simulink model "demo_fengine"  (Simulink 7.4)
settings: Solver=ode45  StartTime=0.0  StopTime=10.0  RelTol=1e-3
undefined symbols (not defined in the file): pfb_coeff_gen_calc
libraries: xbsIndex_r4 x5167, casper_library x437, isi_correlator_lib x15, xps_library x7
(part 1 of 50)
settings Model: Creator=wjm  LastModifiedBy=wjm

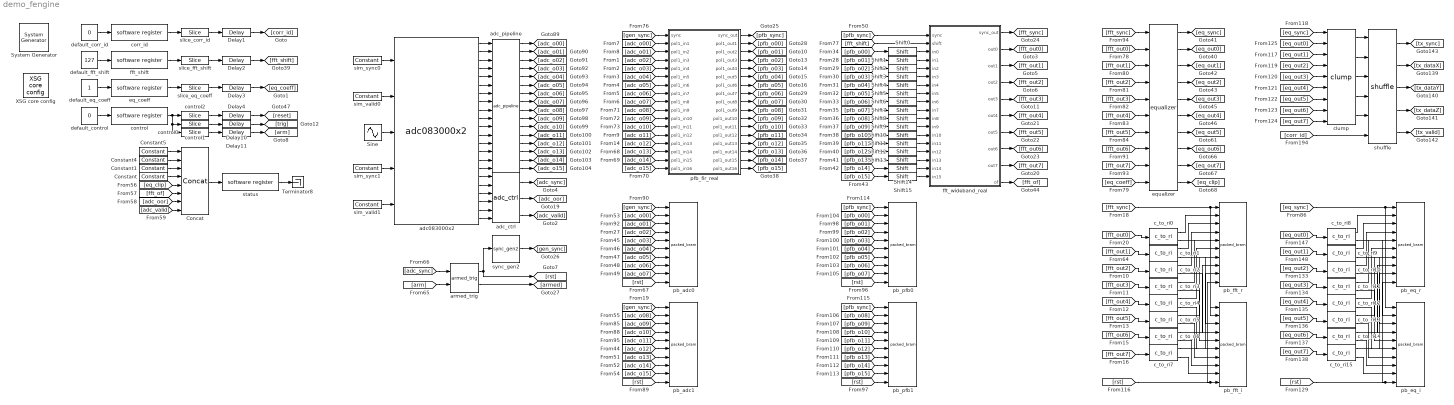
== system demo_fengine ==
  " System Generator" : xbsIndex_r4/ System Generator  Tag=genX  SystemSampleTime=-1  xilinxfamily=virtex5  part=xc5vsx95t  speed=-1  package=ff1136  synthesis_tool=XST  clock_wrapper=Clock Enables  directory=./demo_fengine/sysgen  testbench=off  simulink_period=1  sysclk_period=5  dcm_input_clock_period=10  incr_netlist=off  trim_vbits=Everywhere in SubSystem  dbl_ovrd=According to Block Masks  core_generation=According to Block Masks  run_coregen=off  deprecated_control=off  eval_field=0  block_type=sysgen
      settings: block_version=11.3
  Concat : xbsIndex_r4/Concat  num_inputs=8  dbl_ovrd=off  block_type=concat
      settings: block_version=10.1.3
  Constant{,1,4..5} (4 alike) : xbsIndex_r4/Constant  arith_type=Boolean  const=0  n_bits=1  bin_pt=0  explicit_period=on  period=1  equ=P=C  opselect=C  inp2=PCIN>>17  opr=+  inp1=P  carry=CIN  dbl_ovrd=off  block_type=constant
      settings: block_version=10.1.1
  Delay{1..4,10..11} (6 alike) : xbsIndex_r4/Delay  en=off  latency=1  dbl_ovrd=off  reg_retiming=on  block_type=delay
  Shift{0..15} (16 alike) : xbsIndex_r4/Shift  shift_dir=Right  shift_bits=1  en=off  latency=0  precision=Full  arith_type="Signed  (2's comp)"  n_bits=16  bin_pt=14  quantization=Truncate  overflow=Wrap  dbl_ovrd=off  block_type=shift
  Sine : Sin  Amplitude=.9  Bias=0  Frequency=37.5*(2*pi/200)  Phase=0  Samples=10  SampleTime=1/16  Offset=0
  Terminator8 : Terminator
  XSG core config : xps_library/XSG core config  Tag=xps:xsg  SystemSampleTime=-1  hw_sys=ROACH:sx95t  ibob_linux=off  clk_src=adc0_clk  iBOB_clk_src=sys_clk  BEE2_ctrl_clk_src=sys_clk  BEE2_usr_clk_src=sys_clk  ROACH_clk_src=adc0_clk  CORR_clk_src=sys_clk  gpio_clk_io_group=iBOB:sma  gpio_clk_bit_index=0  clk_rate=200  sample_period=1  synthesis_tool=XST  mpc_type=powerpc440_ext
  adc083000x2 : xps_library/adc083000x2  Tag=xps:adc083000x2  SystemSampleTime=-1  use_adc0=on  use_adc1=on  demux_adc=off  clock_sync=on  verbose=off  using_ctrl=off  adc_clk_rate=800  sample_period=1  bit_width=8
  adc_ctrl : isi_correlator_lib/adc_ctrl  SystemSampleTime=-1
  adc_pipeline : isi_correlator_lib/adc_pipeline  SystemSampleTime=-1  latency=1
  armed_trig : isi_correlator_lib/armed_trig  SystemSampleTime=-1
  c_to_ri{0..7} (8 alike) : casper_library/Misc/c_to_ri  SystemSampleTime=-1  n_bits=18  bin_pt=17
      settings: AttributesFormatString=18_17 r/i
  c_to_ri{8..15} (8 alike) : casper_library/Misc/c_to_ri  SystemSampleTime=-1  n_bits=4  bin_pt=3
      settings: AttributesFormatString=4_3 r/i
  clump : isi_correlator_lib/clump  SystemSampleTime=-1  latency=1
  control : xps_library/software register  Tag=xps:sw_reg  SystemSampleTime=-1  io_dir=From Processor  arith_type=Unsigned  bitwidth=32  bin_pt=0  sample_period=1  gw_name=demo_fengine_control_user_data_out
  control0 : xbsIndex_r4/Slice  nbits=1  boolean_output=on  mode=Lower Bit Location + Width  bit1=0  base1=MSB of Input  bit0=0  base0=LSB of Input  dbl_ovrd=off  block_type=slice
      settings: infoedit="Extracts a given range of bits from each input sample and presents it at the output.  The output type is ordinarily unsigned with binary point at zero, but can be Boolean when the slice is one bit wide.<P><P>Hardware notes: In hardware this block costs nothing."  block_version=10.1.3
  control1 : xbsIndex_r4/Slice  nbits=1  boolean_output=on  mode=Lower Bit Location + Width  bit1=0  base1=MSB of Input  bit0=1  base0=LSB of Input  dbl_ovrd=off  block_type=slice
      settings: infoedit="Extracts a given range of bits from each input sample and presents it at the output.  The output type is ordinarily unsigned with binary point at zero, but can be Boolean when the slice is one bit wide.<P><P>Hardware notes: In hardware this block costs nothing."  block_version=10.1.3
  control2 : xbsIndex_r4/Slice  nbits=1  boolean_output=on  mode=Lower Bit Location + Width  bit1=0  base1=MSB of Input  bit0=2  base0=LSB of Input  dbl_ovrd=off  block_type=slice
      settings: infoedit="Extracts a given range of bits from each input sample and presents it at the output.  The output type is ordinarily unsigned with binary point at zero, but can be Boolean when the slice is one bit wide.<P><P>Hardware notes: In hardware this block costs nothing."  block_version=10.1.3
  corr_id : xps_library/software register  Tag=xps:sw_reg  SystemSampleTime=-1  io_dir=From Processor  arith_type=Unsigned  bitwidth=32  bin_pt=0  sample_period=1  gw_name=demo_fengine_corr_id_user_data_out
  default_control : Constant  Value=0  OutDataType=sfix(16)  OutScaling=2^0  SampleTime=1
  default_corr_id : Constant  Value=0  OutDataType=sfix(16)  OutScaling=2^0  SampleTime=1
  default_eq_coeff : Constant  OutDataType=sfix(16)  OutScaling=2^0  SampleTime=1
  default_fft_shift : Constant  Value=127  OutDataType=sfix(16)  OutScaling=2^0  SampleTime=1
  eq_coeff : xps_library/software register  Tag=xps:sw_reg  SystemSampleTime=-1  io_dir=From Processor  arith_type=Unsigned  bitwidth=32  bin_pt=0  sample_period=1  gw_name=demo_fengine_eq_coeff_user_data_out
  equalizer : isi_correlator_lib/equalizer  SystemSampleTime=-1  in_bit_width=18  out_bit_width=4
  fft_shift : xps_library/software register  Tag=xps:sw_reg  SystemSampleTime=-1  io_dir=From Processor  arith_type=Unsigned  bitwidth=32  bin_pt=0  sample_period=1  gw_name=demo_fengine_fft_shift_user_data_out
  fft_wideband_real : Subsystem  AncestorBlock=casper_library/FFTs/fft_wideband_real  mask(fft_wideband_real){FFTSize=7, input_bit_width=18, coeff_bit_width=18, n_inputs=4, quantization=Truncate, overflow=Wrap, add_latency=1, mult_latency=3, bram_latency=3, arch=Virtex5, opt_target=Multipliers, coeffs_bit_limit=8, delays_bit_limit=9, specify_mult=on, mult_spec=[1 1 1 1 1 1 1], dsp48_adders=on}
      mask prompts: FFTSize "Size of FFT: (2^?)"; input_bit_width "Input/Output Bit Width"; coeff_bit_width "Coefficient Bit Width"; n_inputs "Number of Simultaneous Inputs: (2^?)"; quantization "Quantization Behavior"; overflow "Overflow Behavior"; add_latency "Add Latency"; mult_latency "Mult Latency"; bram_latency "BRAM Latency"; arch "Architecture"; opt_target "Optimisation Target"; coeffs_bit_limit "Number bits above which to store stage's coefficients in BRAM (2^? bits)"; delays_bit_limit "Number bits above which to store stage's delays in BRAM (2^? bits)"; specify_mult "Specify multiplier use (behavioral HDL if not specified)"; mult_spec "Multiplier specification (0=core, 1=embedded, 2=behavioural) (left=1st stage)"; dsp48_adders "Use DSP48s for adders"
      mask init:
      | fft_wideband_real_init(gcb,...
      |     'FFTSize', FFTSize,...
      |     'input_bit_width', input_bit_width,...
      |     'coeff_bit_width', coeff_bit_width,...
      |     'n_inputs', n_inputs,...
      |     'quantization', quantization,...
      |     'overflow', overflow,...
      |     'add_latency', add_latency,...
      |     'mult_latency', mult_latency,...
      |     'bram_latency', bram_latency,...
      |     'arch', arch, ...
      |     'opt_target', opt_target, ...
      |     'coeffs_bit_limit', coeffs_bit_limit, ...
      |     'delays_bit_limit', delays_bit_limit, ...    
      |     'specify_mult', specify_mult,...
      |     'mult_spec', mult_spec, ...
      |     'dsp48_adders', dsp48_adders);
      settings: AttributesFormatString=FFTSize=7
      mask Help: eval('xlWeb([getenv(''MLIB_ROOT''), ''\doc\htmlblocks\sp_fft_wideband_real\sp_fft_wideband_real.html''])')
      mask StyleString: edit,edit,edit,edit,popup(Truncate|Round  (unbiased: +/- Inf)|Round  (unbiased: Even Values)),popup(Wrap|Saturate|Error),edit,edit,edit,popup(Virtex2Pro|Virtex5),popup(Logic|Multipliers),edit,edit,checkbox,edit,checkbox
      mask CallbackString:
      | % fft_size = eval(get_param(gcb, 'FFTSize'));
      | % old_vec = eval(get_param(gcb, 'mult_spec'));
      | 
      | % vec = 2.*ones(1,fft_size);
      | % old_size = length(old_vec);
      | 
      | % if(old_size <= fft_size),
      | %    size = old_size;
      | % else,
      | %     size = fft_size;
      | % end
      | 
      | % for n = 1:size, vec(n) = old_vec(n); end
      | % set_param(gcb, 'mult_spec', mat2str(vec));|||||||||||||specify_mult = get_param(gcb,'specify_mult');
      | visibs = get_param(gcb, 'MaskVisibilities');
      | enables = get_param(gcb, 'MaskEnables');
      | enables{15} = 'on';
      | if( strcmp(specify_mult,'off')), visibs{15} = 'off';
      | else, visibs{15} = 'on';
      | end
      | set_param(gcb, 'MaskEnables', enables);
      | set_param(gcb, 'MaskVisibilities', visibs);
      | |% vec = eval( get_param(gcb, 'mult_spec'));
      | % vec_size = length(vec);
      | % fft_size = eval( get_param(gcb, 'FFTSize'));
      | % new_vec = 2.*ones(1, fft_size);
      | 
      | % if(vec_size <= fft_size), size = vec_size;
      | % else size = fft_size;
      | % end
      | 
      | % for n=1:size
      | %     new_vec(n) = vec(n);
      | % end
      | % set_param(gcb, 'mult_spec', mat2str(new_vec));|
  pb_adc0 : isi_correlator_lib/packed_bram  SystemSampleTime=-1  window_length=32  bit_width=8  fanin_latency=3
      settings: AttributesFormatString=size = 1024B
  pb_adc1 : isi_correlator_lib/packed_bram  SystemSampleTime=-1  window_length=32  bit_width=8  fanin_latency=3
      settings: AttributesFormatString=size = 1024B
  pb_eq_i : isi_correlator_lib/packed_bram  SystemSampleTime=-1  window_length=8  bit_width=4  fanin_latency=3
      settings: AttributesFormatString=size = 256B
  pb_eq_r : isi_correlator_lib/packed_bram  SystemSampleTime=-1  window_length=8  bit_width=4  fanin_latency=3
      settings: AttributesFormatString=size = 256B
  pb_fft_i : isi_correlator_lib/packed_bram  SystemSampleTime=-1  window_length=8  bit_width=18  fanin_latency=3
      settings: AttributesFormatString=size = 256B
  pb_fft_r : isi_correlator_lib/packed_bram  SystemSampleTime=-1  window_length=8  bit_width=18  fanin_latency=3
      settings: AttributesFormatString=size = 256B
  pb_pfb0 : isi_correlator_lib/packed_bram  SystemSampleTime=-1  window_length=8  bit_width=18  fanin_latency=3
      settings: AttributesFormatString=size = 256B
  pb_pfb1 : isi_correlator_lib/packed_bram  SystemSampleTime=-1  window_length=8  bit_width=18  fanin_latency=3
      settings: AttributesFormatString=size = 256B
  pfb_fir_real : Subsystem  AncestorBlock=casper_library/PFBs/pfb_fir_real  mask(pfb_fir_real){PFBSize=7, TotalTaps=4, WindowType=hamming, n_inputs=4, MakeBiplex=0, BitWidthIn=8, BitWidthOut=18, CoeffBitWidth=18, CoeffDistMem=0, add_latency=2, mult_latency=3, bram_latency=2, quantization=Truncate, fwidth=1, specify_mult=on, mult_spec=[1 1 1 1], behavioral=on}
      mask prompts: PFBSize "Size of PFB: (2^? pnts)"; TotalTaps "Total Number of Taps:"; WindowType "Windowing Function:"; n_inputs "Number of Simultaneous Inputs: (2^?)"; MakeBiplex "Make Biplex"; BitWidthIn "Input Bitwidth:"; BitWidthOut "Output Bitwidth:"; CoeffBitWidth "Coefficient Bitwidth:"; CoeffDistMem "Use Distributed Memory for Coeffs"; add_latency "Add Latency"; mult_latency "Mult Latency"; bram_latency "BRAM Latency"; quantization "Quantization Behavior"; fwidth "Bin Width Scaling (normal=1)"; specify_mult "Specify multiplier use (embedded if not specified)"; mult_spec "Multiplier specification (0=core, 1=embedded, 2=behavioural) (left=1st tap)"; behavioral "Use behavioral HDL for adder trees"
      mask init:
      | pfb_fir_real_init(gcb,...
      |     'PFBSize', PFBSize,...
      |     'TotalTaps', TotalTaps,...
      |     'WindowType', WindowType,...
      |     'n_inputs', n_inputs,...
      |     'MakeBiplex', MakeBiplex,...
      |     'BitWidthIn', BitWidthIn,...
      |     'BitWidthOut', BitWidthOut,...
      |     'CoeffBitWidth', CoeffBitWidth,...
      |     'CoeffDistMem', CoeffDistMem,...
      |     'add_latency', add_latency,...
      |     'mult_latency', mult_latency,...
      |     'bram_latency', bram_latency,...
      |     'quantization', quantization,...
      |     'fwidth', fwidth,...
      |     'specify_mult', specify_mult,...
      |     'mult_spec', mult_spec,...
      |     'behavioral', behavioral);
      settings: AttributesFormatString=taps=4, add_latency=2
      mask Help: eval('xlWeb([getenv(''MLIB_ROOT''), ''\doc\htmlblocks\sp_pfb_fir_real\sp_pfb_fir_real.html''])')
      mask StyleString: edit,edit,popup(bartlett|barthannwin|blackman|blackmanharris|bohamwin|chebwin|flattopwin|gausswin|hamming|hann|kaiser|nuttallwin|parzenwin|rectwin|tukeywin|triang|userwindow),edit,edit,edit,edit,edit,edit,edit,edit,edit,popup(Truncate|Round  (unbiased: +/- Inf)|Round  (unbiased: Even Values)),edit,checkbox,edit,checkbox
      mask CallbackString:
      | |num_taps = eval(get_param(gcb, 'TotalTaps'));
      | old_vec = eval(get_param(gcb, 'mult_spec'));
      | 
      | vec = ones(1,num_taps);
      | old_size = length(old_vec);
      | 
      | if(old_size <= num_taps),
      |     size = old_size;
      | else,
      |     size = num_taps;
      | end
      | 
      | for n = 1:size, vec(n) = old_vec(n); end
      | set_param(gcb, 'mult_spec', mat2str(vec));|||||||||||||thisVariable = 'specify_mult';
      | affectedVars = {'mult_spec'};
      | 
      | thisBlock = gcb();
      | thisVarState = get_param(thisBlock, thisVariable);
      | maskNames = get_param(thisBlock, 'MaskNames');
      | maskEnables = get_param(thisBlock, 'MaskEnables');
      | 
      | for varName = affectedVars
      |     varIndex = find(ismember(maskNames, varName));
      |     maskEnables{varIndex} = thisVarState;
      | end
      | 
      | set_param(thisBlock, 'MaskEnables', maskEnables);|vec = eval( get_param(gcb, 'mult_spec'));
      | vec_size = length(vec);
      | num_taps = eval( get_param(gcb, 'TotalTaps'));
      | new_vec = ones(1, num_taps);
      | 
      | if(vec_size <= num_taps), size = vec_size;
      | else size = num_taps;
      | end
      | 
      | for n=1:size
      |     new_vec(n) = vec(n);
      | end
      | set_param(gcb, 'mult_spec', mat2str(new_vec));|
  shuffle : isi_correlator_lib/shuffle  SystemSampleTime=-1  latency=3
  sim_sync0 : xbsIndex_r4/Constant  arith_type=Unsigned  const=0  n_bits=4  bin_pt=0  explicit_period=on  period=1  equ=P=C  opselect=C  inp2=PCIN>>17  opr=+  inp1=P  carry=CIN  dbl_ovrd=off  block_type=constant
      settings: block_version=10.1.1
  sim_sync1 : xbsIndex_r4/Constant  arith_type=Unsigned  const=0  n_bits=4  bin_pt=0  explicit_period=on  period=1  equ=P=C  opselect=C  inp2=PCIN>>17  opr=+  inp1=P  carry=CIN  dbl_ovrd=off  block_type=constant
      settings: block_version=10.1.1
  sim_valid0 : xbsIndex_r4/Constant  arith_type=Unsigned  const=0  n_bits=1  bin_pt=0  explicit_period=on  period=1  equ=P=C  opselect=C  inp2=PCIN>>17  opr=+  inp1=P  carry=CIN  dbl_ovrd=off  block_type=constant
      settings: block_version=10.1.1
  sim_valid1 : xbsIndex_r4/Constant  arith_type=Unsigned  const=0  n_bits=1  bin_pt=0  explicit_period=on  period=1  equ=P=C  opselect=C  inp2=PCIN>>17  opr=+  inp1=P  carry=CIN  dbl_ovrd=off  block_type=constant
      settings: block_version=10.1.1
  slice_corr_id : xbsIndex_r4/Slice  nbits=2  boolean_output=off  mode=Lower Bit Location + Width  bit1=0  base1=MSB of Input  bit0=0  base0=LSB of Input  dbl_ovrd=off  block_type=slice
      settings: infoedit="Extracts a given range of bits from each input sample and presents it at the output.  The output type is ordinarily unsigned with binary point at zero, but can be Boolean when the slice is one bit wide.<P><P>Hardware notes: In hardware this block costs nothing."  block_version=10.1.3
  slice_eq_coeff : xbsIndex_r4/Slice  nbits=14  boolean_output=off  mode=Lower Bit Location + Width  bit1=0  base1=MSB of Input  bit0=0  base0=LSB of Input  dbl_ovrd=off  block_type=slice
      settings: block_version=10.1.3
  slice_fft_shift : xbsIndex_r4/Slice  nbits=7  boolean_output=off  mode=Lower Bit Location + Width  bit1=0  base1=MSB of Input  bit0=0  base0=LSB of Input  dbl_ovrd=off  block_type=slice
      settings: infoedit="Extracts a given range of bits from each input sample and presents it at the output.  The output type is ordinarily unsigned with binary point at zero, but can be Boolean when the slice is one bit wide.<P><P>Hardware notes: In hardware this block costs nothing."  block_version=10.1.3
  status : xps_library/software register  Tag=xps:sw_reg  SystemSampleTime=-1  io_dir=To Processor  arith_type=Unsigned  bitwidth=32  bin_pt=0  sample_period=1  gw_name=demo_fengine_status_user_data_in
  sync_gen2 : isi_correlator_lib/sync_gen2  SystemSampleTime=-1
  wires:
  [pfb_sync] -> fft_wideband_real/1(sync)
  fft_wideband_real/2(out0) -> [fft_out0]
  fft_wideband_real/3(out1) -> [fft_out1]
  fft_wideband_real/4(out2) -> [fft_out2]
  fft_wideband_real/5(out3) -> [fft_out3]
  fft_wideband_real/6(out4) -> [fft_out4]
  fft_wideband_real/7(out5) -> [fft_out5]
  fft_wideband_real/8(out6) -> [fft_out6]
  fft_wideband_real/9(out7) -> [fft_out7]
  [adc_o00] -> pfb_fir_real/2(pol1_in1)
  [adc_o01] -> pfb_fir_real/3(pol1_in2)
  [adc_o02] -> pfb_fir_real/4(pol1_in3)
  [adc_o03] -> pfb_fir_real/5(pol1_in4)
  [adc_o04] -> pfb_fir_real/6(pol1_in5)
  [adc_o05] -> pfb_fir_real/7(pol1_in6)
  [adc_o06] -> pfb_fir_real/8(pol1_in7)
  [adc_o07] -> pfb_fir_real/9(pol1_in8)
  [adc_o08] -> pfb_fir_real/10(pol1_in9)
  [adc_o09] -> pfb_fir_real/11(pol1_in10)
  [adc_o10] -> pfb_fir_real/12(pol1_in11)
  [adc_o11] -> pfb_fir_real/13(pol1_in12)
  [adc_o12] -> pfb_fir_real/14(pol1_in13)
  [adc_o13] -> pfb_fir_real/15(pol1_in14)
  [adc_o14] -> pfb_fir_real/16(pol1_in15)
  [adc_o15] -> pfb_fir_real/17(pol1_in16)
  pfb_fir_real/1(sync_out) -> [pfb_sync]
  pfb_fir_real/2(pol1_out1) -> [pfb_o00]
  pfb_fir_real/3(pol1_out2) -> [pfb_o01]
  pfb_fir_real/4(pol1_out3) -> [pfb_o02]
  pfb_fir_real/5(pol1_out4) -> [pfb_o03]
  pfb_fir_real/6(pol1_out5) -> [pfb_o04]
  pfb_fir_real/7(pol1_out6) -> [pfb_o05]
  pfb_fir_real/8(pol1_out7) -> [pfb_o06]
  pfb_fir_real/9(pol1_out8) -> [pfb_o07]
  pfb_fir_real/10(pol1_out9) -> [pfb_o08]
  pfb_fir_real/11(pol1_out10) -> [pfb_o09]
  pfb_fir_real/12(pol1_out11) -> [pfb_o10]
  pfb_fir_real/13(pol1_out12) -> [pfb_o11]
  pfb_fir_real/14(pol1_out13) -> [pfb_o12]
  pfb_fir_real/15(pol1_out14) -> [pfb_o13]
  pfb_fir_real/16(pol1_out15) -> [pfb_o14]
  equalizer/2 -> [eq_out0]
  equalizer/3 -> [eq_out1]
  equalizer/4 -> [eq_out2]
  equalizer/5 -> [eq_out3]
  equalizer/6 -> [eq_out4]
  equalizer/7 -> [eq_out5]
  equalizer/8 -> [eq_out6]
  equalizer/9 -> [eq_out7]
  [fft_sync] -> equalizer/1
  [fft_out0] -> equalizer/2
  [fft_out1] -> equalizer/3
  [fft_out2] -> equalizer/4
  [fft_out3] -> equalizer/5
  [fft_out4] -> equalizer/6
  [fft_out5] -> equalizer/7
  [fft_out6] -> equalizer/8
  [fft_out7] -> equalizer/9
  fft_wideband_real/1(sync_out) -> [fft_sync]
  [fft_shift] -> fft_wideband_real/2(shift)
  equalizer/1 -> [eq_sync]
  pfb_fir_real/17(pol1_out16) -> [pfb_o15]
  [eq_coeff] -> equalizer/10
  equalizer/10 -> [eq_clip]
  fft_wideband_real/10(of) -> [fft_of]
  [eq_out0] -> clump/2
  [eq_out1] -> clump/3
  [eq_out2] -> clump/4
  [eq_out3] -> clump/5
  [eq_out4] -> clump/6
  [eq_out5] -> clump/7
  [eq_out6] -> clump/8
  [eq_out7] -> clump/9
  shuffle/1 -> [tx_sync]
  shuffle/2 -> [tx_dataX]
  shuffle/3 -> [tx_dataY]
  shuffle/4 -> [tx_dataZ]
  shuffle/5 -> [tx_valid]
  [eq_sync] -> clump/1
  clump/1 -> shuffle/1
  clump/2 -> shuffle/2
  clump/3 -> shuffle/3
  clump/4 -> shuffle/4
  clump/5 -> shuffle/5
  [corr_id] -> shuffle/6
  adc_ctrl/1 -> [adc_sync]
  adc_ctrl/2 -> [adc_oor]
  adc_ctrl/3 -> [adc_valid]
  [arm] -> armed_trig/2
  armed_trig/1 -> sync_gen2/1, [rst]
  armed_trig/2 -> [armed]
  sync_gen2/1 -> [gen_sync]
  [gen_sync] -> pfb_fir_real/1(sync)
  adc_pipeline/1 -> [adc_o00]
  adc_pipeline/2 -> [adc_o01]
  adc_pipeline/3 -> [adc_o02]
  adc_pipeline/4 -> [adc_o03]
  adc_pipeline/5 -> [adc_o04]
  adc_pipeline/6 -> [adc_o05]
  adc_pipeline/7 -> [adc_o06]
  adc_pipeline/8 -> [adc_o07]
  adc_pipeline/9 -> [adc_o08]
  adc_pipeline/10 -> [adc_o09]
  adc_pipeline/11 -> [adc_o10]
  adc_pipeline/12 -> [adc_o11]
  adc_pipeline/13 -> [adc_o12]
  adc_pipeline/14 -> [adc_o13]
  adc_pipeline/15 -> [adc_o14]
  adc_pipeline/16 -> [adc_o15]
  Shift0/1 -> fft_wideband_real/3(in0)
  Shift1/1 -> fft_wideband_real/4(in1)
  Shift2/1 -> fft_wideband_real/5(in2)
  Shift3/1 -> fft_wideband_real/6(in3)
  Shift4/1 -> fft_wideband_real/7(in4)
  Shift5/1 -> fft_wideband_real/8(in5)
  Shift6/1 -> fft_wideband_real/9(in6)
  Shift7/1 -> fft_wideband_real/10(in7)
  Shift8/1 -> fft_wideband_real/11(in8)
  Shift9/1 -> fft_wideband_real/12(in9)
  Shift10/1 -> fft_wideband_real/13(in10)
  Shift11/1 -> fft_wideband_real/14(in11)
  Shift12/1 -> fft_wideband_real/15(in12)
  Shift13/1 -> fft_wideband_real/16(in13)
  Shift14/1 -> fft_wideband_real/17(in14)
  Shift15/1 -> fft_wideband_real/18(in15)
  [pfb_o00] -> Shift0/1
  [pfb_o01] -> Shift1/1
  [pfb_o02] -> Shift2/1
  [pfb_o03] -> Shift3/1
  [pfb_o04] -> Shift4/1
  [pfb_o05] -> Shift5/1
  [pfb_o06] -> Shift6/1
  [pfb_o07] -> Shift7/1
  [pfb_o08] -> Shift8/1
  [pfb_o09] -> Shift9/1
  [pfb_o10] -> Shift10/1
  [pfb_o11] -> Shift11/1
  [pfb_o12] -> Shift12/1
  [pfb_o13] -> Shift13/1
  [pfb_o14] -> Shift14/1
  [pfb_o15] -> Shift15/1
  [adc_sync] -> armed_trig/1
  [gen_sync] -> pb_adc0/1
  [adc_o00] -> pb_adc0/2
  [adc_o01] -> pb_adc0/3
  [adc_o02] -> pb_adc0/4
  [adc_o03] -> pb_adc0/5
  [adc_o04] -> pb_adc0/6
  [adc_o05] -> pb_adc0/7
  [adc_o06] -> pb_adc0/8
  [adc_o07] -> pb_adc0/9
  [gen_sync] -> pb_adc1/1
  [adc_o08] -> pb_adc1/2
  [adc_o09] -> pb_adc1/3
  [adc_o10] -> pb_adc1/4
  [adc_o11] -> pb_adc1/5
  [adc_o12] -> pb_adc1/6
  [adc_o13] -> pb_adc1/7
  [adc_o14] -> pb_adc1/8
  [adc_o15] -> pb_adc1/9
  [rst] -> pb_adc0/10
  [rst] -> pb_adc1/10
  [pfb_sync] -> pb_pfb1/1
  [pfb_o08] -> pb_pfb1/2
  [pfb_o09] -> pb_pfb1/3
  [pfb_o10] -> pb_pfb1/4
  [pfb_o11] -> pb_pfb1/5
  [pfb_o12] -> pb_pfb1/6
  [pfb_o13] -> pb_pfb1/7
  [pfb_o14] -> pb_pfb1/8
  [pfb_o15] -> pb_pfb1/9
  [rst] -> pb_pfb1/10
  [pfb_sync] -> pb_pfb0/1
  [pfb_o00] -> pb_pfb0/2
  [pfb_o01] -> pb_pfb0/3
  [pfb_o02] -> pb_pfb0/4
  [pfb_o03] -> pb_pfb0/5
  [pfb_o04] -> pb_pfb0/6
  [pfb_o05] -> pb_pfb0/7
  [pfb_o06] -> pb_pfb0/8
  [pfb_o07] -> pb_pfb0/9
  [rst] -> pb_pfb0/10
  [fft_sync] -> pb_fft_r/1, pb_fft_i/1
  [fft_out0] -> c_to_ri0/1
  [fft_out1] -> c_to_ri1/1
  [fft_out2] -> c_to_ri2/1
  [fft_out3] -> c_to_ri3/1
  [fft_out4] -> c_to_ri4/1
  [fft_out5] -> c_to_ri5/1
  [fft_out6] -> c_to_ri6/1
  [fft_out7] -> c_to_ri7/1
  [rst] -> pb_fft_i/10, pb_fft_r/10
  c_to_ri0/1 -> pb_fft_r/2
  c_to_ri1/1 -> pb_fft_r/3
  c_to_ri2/1 -> pb_fft_r/4
  c_to_ri3/1 -> pb_fft_r/5
  c_to_ri4/1 -> pb_fft_r/6
  c_to_ri5/1 -> pb_fft_r/7
  c_to_ri6/1 -> pb_fft_r/8
  c_to_ri7/1 -> pb_fft_r/9
  c_to_ri0/2 -> pb_fft_i/2
  c_to_ri1/2 -> pb_fft_i/3
  c_to_ri2/2 -> pb_fft_i/4
  c_to_ri3/2 -> pb_fft_i/5
  c_to_ri4/2 -> pb_fft_i/6
  c_to_ri5/2 -> pb_fft_i/7
  c_to_ri6/2 -> pb_fft_i/8
  c_to_ri7/2 -> pb_fft_i/9
  [eq_sync] -> pb_eq_r/1, pb_eq_i/1
  [rst] -> pb_eq_i/10, pb_eq_r/10
  c_to_ri8/1 -> pb_eq_r/2
  c_to_ri9/1 -> pb_eq_r/3
  c_to_ri10/1 -> pb_eq_r/4
  c_to_ri11/1 -> pb_eq_r/5
  c_to_ri12/1 -> pb_eq_r/6
  c_to_ri13/1 -> pb_eq_r/7
  c_to_ri14/1 -> pb_eq_r/8
  c_to_ri15/1 -> pb_eq_r/9
  c_to_ri8/2 -> pb_eq_i/2
  c_to_ri9/2 -> pb_eq_i/3
  c_to_ri10/2 -> pb_eq_i/4
  c_to_ri11/2 -> pb_eq_i/5
  c_to_ri12/2 -> pb_eq_i/6
  c_to_ri13/2 -> pb_eq_i/7
  c_to_ri14/2 -> pb_eq_i/8
  c_to_ri15/2 -> pb_eq_i/9
  [eq_out0] -> c_to_ri8/1
  [eq_out1] -> c_to_ri9/1
  [eq_out2] -> c_to_ri10/1
  [eq_out3] -> c_to_ri11/1
  [eq_out4] -> c_to_ri12/1
  [eq_out5] -> c_to_ri13/1
  [eq_out6] -> c_to_ri14/1
  [eq_out7] -> c_to_ri15/1
  default_eq_coeff/1 -> eq_coeff/1
  default_control/1 -> control/1
  eq_coeff/1 -> slice_eq_coeff/1
  default_fft_shift/1 -> fft_shift/1
  fft_shift/1 -> slice_fft_shift/1
  Delay2/1 -> [fft_shift]
  Delay3/1 -> [eq_coeff]
  slice_fft_shift/1 -> Delay2/1
  control2/1 -> Delay4/1
  control1/1 -> Delay10/1
  control0/1 -> Delay11/1
  control/1 -> control2/1, control0/1, control1/1
  Delay4/1 -> [reset]
  Delay10/1 -> [trig]
  Delay11/1 -> [arm]
  slice_eq_coeff/1 -> Delay3/1
  sim_sync0/1 -> adc083000x2/1
  sim_valid0/1 -> adc083000x2/2
  Sine/1 -> adc083000x2/3
  sim_sync1/1 -> adc083000x2/4
  sim_valid1/1 -> adc083000x2/5
  adc083000x2/1 -> adc_pipeline/1
  adc083000x2/2 -> adc_pipeline/2
  adc083000x2/3 -> adc_pipeline/3
  adc083000x2/4 -> adc_pipeline/4
  adc083000x2/5 -> adc_pipeline/5
  adc083000x2/6 -> adc_pipeline/6
  adc083000x2/7 -> adc_pipeline/7
  adc083000x2/8 -> adc_pipeline/8
  adc083000x2/9 -> adc_pipeline/9
  adc083000x2/10 -> adc_pipeline/10
  adc083000x2/11 -> adc_pipeline/11
  adc083000x2/12 -> adc_pipeline/12
  adc083000x2/13 -> adc_pipeline/13
  adc083000x2/14 -> adc_pipeline/14
  adc083000x2/15 -> adc_pipeline/15
  adc083000x2/16 -> adc_pipeline/16
  adc083000x2/17 -> adc_ctrl/1
  adc083000x2/18 -> adc_ctrl/2
  adc083000x2/19 -> adc_ctrl/3
  adc083000x2/20 -> adc_ctrl/4
  adc083000x2/21 -> adc_ctrl/5
  adc083000x2/22 -> adc_ctrl/6
  status/1 -> Terminator8/1
  Constant4/1 -> Concat/2
  Constant1/1 -> Concat/3
  Constant/1 -> Concat/4
  [eq_clip] -> Concat/5
  [fft_of] -> Concat/6
  [adc_oor] -> Concat/7
  [adc_valid] -> Concat/8
  Constant5/1 -> Concat/1
  Concat/1 -> status/1
  Delay1/1 -> [corr_id]
  slice_corr_id/1 -> Delay1/1
  corr_id/1 -> slice_corr_id/1
  default_corr_id/1 -> corr_id/1

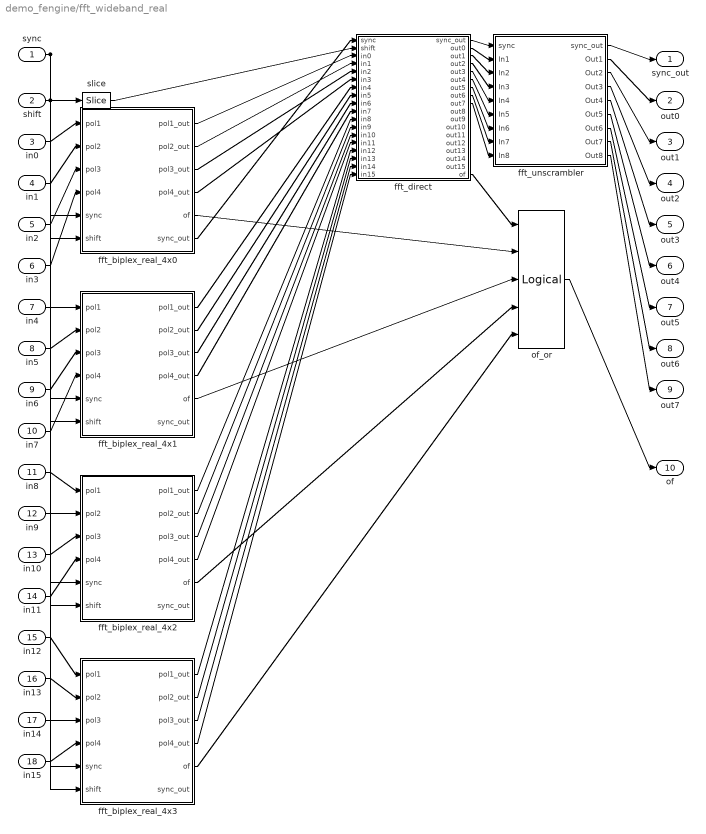
== system demo_fengine/fft_wideband_real ==
  sync : Inport  OutDataType=sfix(16)  OutScaling=2^0
  shift : Inport  Port=2  OutDataType=sfix(16)  OutScaling=2^0
  in0 : Inport  Port=3  OutDataType=sfix(16)  OutScaling=2^0
  in1 : Inport  Port=4  OutDataType=sfix(16)  OutScaling=2^0
  in2 : Inport  Port=5  OutDataType=sfix(16)  OutScaling=2^0
  in3 : Inport  Port=6  OutDataType=sfix(16)  OutScaling=2^0
  in4 : Inport  Port=7
  in5 : Inport  Port=8
  in6 : Inport  Port=9
  in7 : Inport  Port=10
  in8 : Inport  Port=11
  in9 : Inport  Port=12
  in10 : Inport  Port=13
  in11 : Inport  Port=14
  in12 : Inport  Port=15
  in13 : Inport  Port=16
  in14 : Inport  Port=17
  in15 : Inport  Port=18
  fft_biplex_real_4x0 : Subsystem  AncestorBlock=casper_library/FFTs/fft_biplex_real_4x  mask(fft_biplex_real_4x){FFTSize=3, input_bit_width=18, coeff_bit_width=18, quantization=Truncate, overflow=Wrap, add_latency=1, mult_latency=3, bram_latency=3, arch=Virtex5, opt_target=Multipliers, coeffs_bit_limit=8, delays_bit_limit=9, specify_mult=on, mult_spec=[1 1 1], dsp48_adders=on}
      mask prompts: FFTSize "Size of FFT: (2^? pnts)"; input_bit_width "Input/Output Bit Width"; coeff_bit_width "Coefficient Bit Width"; quantization "Quantization Behavior"; overflow "Overflow Behavior"; add_latency "Add Latency"; mult_latency "Mult Latency"; bram_latency "BRAM Latency"; arch "Architecture"; opt_target "Optimisation Target"; coeffs_bit_limit "Number bits above which to store stage's coefficients in BRAM (2^? bits)"; delays_bit_limit "Number bits above which to implement stage's delays in BRAM (2^? bits)"; specify_mult "Specify multiplier use (behavioral HDL if not specified)"; mult_spec "Multiplier specification (0=core, 1=embedded, 2=behavioural) (left=1st stage)"; dsp48_adders "Use DSP48s for adders"
      mask init:
      | fft_biplex_real_4x_init(gcb, ...
      |     'FFTSize', FFTSize, ...
      |     'input_bit_width', input_bit_width, ...
      |     'coeff_bit_width', coeff_bit_width, ...
      |     'quantization', quantization, ...
      |     'overflow', overflow, ...
      |     'add_latency', add_latency, ...
      |     'mult_latency', mult_latency, ...
      |     'bram_latency', bram_latency, ...
      |     'arch', arch, ...
      |     'opt_target', opt_target, ...
      |     'coeffs_bit_limit', coeffs_bit_limit, ...
      |     'delays_bit_limit', delays_bit_limit, ...    
      |     'specify_mult', specify_mult, ...
      |     'mult_spec', mult_spec, ...
      |     'dsp48_adders', dsp48_adders);
      settings: AttributesFormatString=FFTSize=3
      mask Help: eval('xlWeb([getenv(''MLIB_ROOT''), ''\doc\htmlblocks\sp_fft_biplex_real_4x\sp_fft_biplex_real_4x.html''])')
      mask StyleString: edit,edit,edit,popup(Truncate|Round  (unbiased: +/- Inf)|Round  (unbiased: Even Values)),popup(Wrap|Saturate|Error),edit,edit,edit,popup(Virtex2Pro|Virtex5),popup(Logic|Multipliers),edit,edit,checkbox,edit,checkbox
      mask CallbackString:
      | % fft_size = eval(get_param(gcb, 'FFTSize'));
      | % old_vec = eval(get_param(gcb, 'mult_spec'));
      | 
      | % vec = 2.*ones(1,fft_size);
      | % old_size = length(old_vec);
      | 
      | % if(old_size <= fft_size),
      | %     size = old_size;
      | % else,
      | %     size = fft_size;
      | % end
      | 
      | % for n = 1:size, vec(n) = old_vec(n); end
      | % set_param(gcb, 'mult_spec', mat2str(vec));||||||||||||specify_mult = get_param(gcb,'specify_mult');
      | visibs = get_param(gcb, 'MaskVisibilities');
      | enables = get_param(gcb, 'MaskEnables');
      | enables{14} = 'on';
      | if( strcmp(specify_mult,'off')), visibs{14} = 'off';
      | else, visibs{14} = 'on';
      | end
      | set_param(gcb, 'MaskVisibilities', visibs);
      | set_param(gcb, 'MaskEnables', enables);
      | |% vec = eval( get_param(gcb, 'mult_spec'));
      | % vec_size = length(vec);
      | % fft_size = eval( get_param(gcb, 'FFTSize'));
      | % new_vec = 2.*ones(1, fft_size);
      | 
      | % if(vec_size <= fft_size), size = vec_size;
      | % else size = fft_size;
      | % end
      | 
      | % for n=1:size
      | %    new_vec(n) = vec(n);
      | % end
      | % set_param(gcb, 'mult_spec', mat2str(new_vec));|
  fft_biplex_real_4x1 : Subsystem  AncestorBlock=casper_library/FFTs/fft_biplex_real_4x  mask(fft_biplex_real_4x){FFTSize=3, input_bit_width=18, coeff_bit_width=18, quantization=Truncate, overflow=Wrap, add_latency=1, mult_latency=3, bram_latency=3, arch=Virtex5, opt_target=Multipliers, coeffs_bit_limit=8, delays_bit_limit=9, specify_mult=on, mult_spec=[1 1 1], dsp48_adders=on}
      mask prompts: FFTSize "Size of FFT: (2^? pnts)"; input_bit_width "Input/Output Bit Width"; coeff_bit_width "Coefficient Bit Width"; quantization "Quantization Behavior"; overflow "Overflow Behavior"; add_latency "Add Latency"; mult_latency "Mult Latency"; bram_latency "BRAM Latency"; arch "Architecture"; opt_target "Optimisation Target"; coeffs_bit_limit "Number bits above which to store stage's coefficients in BRAM (2^? bits)"; delays_bit_limit "Number bits above which to implement stage's delays in BRAM (2^? bits)"; specify_mult "Specify multiplier use (behavioral HDL if not specified)"; mult_spec "Multiplier specification (0=core, 1=embedded, 2=behavioural) (left=1st stage)"; dsp48_adders "Use DSP48s for adders"
      mask init: same as demo_fengine/fft_wideband_real/fft_biplex_real_4x0
      settings: AttributesFormatString=FFTSize=3
      mask Help: eval('xlWeb([getenv(''MLIB_ROOT''), ''\doc\htmlblocks\sp_fft_biplex_real_4x\sp_fft_biplex_real_4x.html''])')
      mask StyleString: edit,edit,edit,popup(Truncate|Round  (unbiased: +/- Inf)|Round  (unbiased: Even Values)),popup(Wrap|Saturate|Error),edit,edit,edit,popup(Virtex2Pro|Virtex5),popup(Logic|Multipliers),edit,edit,checkbox,edit,checkbox
      mask CallbackString:
      | % fft_size = eval(get_param(gcb, 'FFTSize'));
      | % old_vec = eval(get_param(gcb, 'mult_spec'));
      | 
      | % vec = 2.*ones(1,fft_size);
      | % old_size = length(old_vec);
      | 
      | % if(old_size <= fft_size),
      | %     size = old_size;
      | % else,
      | %     size = fft_size;
      | % end
      | 
      | % for n = 1:size, vec(n) = old_vec(n); end
      | % set_param(gcb, 'mult_spec', mat2str(vec));||||||||||||specify_mult = get_param(gcb,'specify_mult');
      | visibs = get_param(gcb, 'MaskVisibilities');
      | enables = get_param(gcb, 'MaskEnables');
      | enables{14} = 'on';
      | if( strcmp(specify_mult,'off')), visibs{14} = 'off';
      | else, visibs{14} = 'on';
      | end
      | set_param(gcb, 'MaskVisibilities', visibs);
      | set_param(gcb, 'MaskEnables', enables);
      | |% vec = eval( get_param(gcb, 'mult_spec'));
      | % vec_size = length(vec);
      | % fft_size = eval( get_param(gcb, 'FFTSize'));
      | % new_vec = 2.*ones(1, fft_size);
      | 
      | % if(vec_size <= fft_size), size = vec_size;
      | % else size = fft_size;
      | % end
      | 
      | % for n=1:size
      | %    new_vec(n) = vec(n);
      | % end
      | % set_param(gcb, 'mult_spec', mat2str(new_vec));|
  fft_biplex_real_4x2 : Subsystem  AncestorBlock=casper_library/FFTs/fft_biplex_real_4x  mask(fft_biplex_real_4x){FFTSize=3, input_bit_width=18, coeff_bit_width=18, quantization=Truncate, overflow=Wrap, add_latency=1, mult_latency=3, bram_latency=3, arch=Virtex5, opt_target=Multipliers, coeffs_bit_limit=8, delays_bit_limit=9, specify_mult=on, mult_spec=[1 1 1], dsp48_adders=on}
      mask prompts: FFTSize "Size of FFT: (2^? pnts)"; input_bit_width "Input/Output Bit Width"; coeff_bit_width "Coefficient Bit Width"; quantization "Quantization Behavior"; overflow "Overflow Behavior"; add_latency "Add Latency"; mult_latency "Mult Latency"; bram_latency "BRAM Latency"; arch "Architecture"; opt_target "Optimisation Target"; coeffs_bit_limit "Number bits above which to store stage's coefficients in BRAM (2^? bits)"; delays_bit_limit "Number bits above which to implement stage's delays in BRAM (2^? bits)"; specify_mult "Specify multiplier use (behavioral HDL if not specified)"; mult_spec "Multiplier specification (0=core, 1=embedded, 2=behavioural) (left=1st stage)"; dsp48_adders "Use DSP48s for adders"
      mask init: same as demo_fengine/fft_wideband_real/fft_biplex_real_4x0
      settings: AttributesFormatString=FFTSize=3
      mask Help: eval('xlWeb([getenv(''MLIB_ROOT''), ''\doc\htmlblocks\sp_fft_biplex_real_4x\sp_fft_biplex_real_4x.html''])')
      mask StyleString: edit,edit,edit,popup(Truncate|Round  (unbiased: +/- Inf)|Round  (unbiased: Even Values)),popup(Wrap|Saturate|Error),edit,edit,edit,popup(Virtex2Pro|Virtex5),popup(Logic|Multipliers),edit,edit,checkbox,edit,checkbox
      mask CallbackString:
      | % fft_size = eval(get_param(gcb, 'FFTSize'));
      | % old_vec = eval(get_param(gcb, 'mult_spec'));
      | 
      | % vec = 2.*ones(1,fft_size);
      | % old_size = length(old_vec);
      | 
      | % if(old_size <= fft_size),
      | %     size = old_size;
      | % else,
      | %     size = fft_size;
      | % end
      | 
      | % for n = 1:size, vec(n) = old_vec(n); end
      | % set_param(gcb, 'mult_spec', mat2str(vec));||||||||||||specify_mult = get_param(gcb,'specify_mult');
      | visibs = get_param(gcb, 'MaskVisibilities');
      | enables = get_param(gcb, 'MaskEnables');
      | enables{14} = 'on';
      | if( strcmp(specify_mult,'off')), visibs{14} = 'off';
      | else, visibs{14} = 'on';
      | end
      | set_param(gcb, 'MaskVisibilities', visibs);
      | set_param(gcb, 'MaskEnables', enables);
      | |% vec = eval( get_param(gcb, 'mult_spec'));
      | % vec_size = length(vec);
      | % fft_size = eval( get_param(gcb, 'FFTSize'));
      | % new_vec = 2.*ones(1, fft_size);
      | 
      | % if(vec_size <= fft_size), size = vec_size;
      | % else size = fft_size;
      | % end
      | 
      | % for n=1:size
      | %    new_vec(n) = vec(n);
      | % end
      | % set_param(gcb, 'mult_spec', mat2str(new_vec));|
  fft_biplex_real_4x3 : Subsystem  AncestorBlock=casper_library/FFTs/fft_biplex_real_4x  mask(fft_biplex_real_4x){FFTSize=3, input_bit_width=18, coeff_bit_width=18, quantization=Truncate, overflow=Wrap, add_latency=1, mult_latency=3, bram_latency=3, arch=Virtex5, opt_target=Multipliers, coeffs_bit_limit=8, delays_bit_limit=9, specify_mult=on, mult_spec=[1 1 1], dsp48_adders=on}
      mask prompts: FFTSize "Size of FFT: (2^? pnts)"; input_bit_width "Input/Output Bit Width"; coeff_bit_width "Coefficient Bit Width"; quantization "Quantization Behavior"; overflow "Overflow Behavior"; add_latency "Add Latency"; mult_latency "Mult Latency"; bram_latency "BRAM Latency"; arch "Architecture"; opt_target "Optimisation Target"; coeffs_bit_limit "Number bits above which to store stage's coefficients in BRAM (2^? bits)"; delays_bit_limit "Number bits above which to implement stage's delays in BRAM (2^? bits)"; specify_mult "Specify multiplier use (behavioral HDL if not specified)"; mult_spec "Multiplier specification (0=core, 1=embedded, 2=behavioural) (left=1st stage)"; dsp48_adders "Use DSP48s for adders"
      mask init: same as demo_fengine/fft_wideband_real/fft_biplex_real_4x0
      settings: AttributesFormatString=FFTSize=3
      mask Help: eval('xlWeb([getenv(''MLIB_ROOT''), ''\doc\htmlblocks\sp_fft_biplex_real_4x\sp_fft_biplex_real_4x.html''])')
      mask StyleString: edit,edit,edit,popup(Truncate|Round  (unbiased: +/- Inf)|Round  (unbiased: Even Values)),popup(Wrap|Saturate|Error),edit,edit,edit,popup(Virtex2Pro|Virtex5),popup(Logic|Multipliers),edit,edit,checkbox,edit,checkbox
      mask CallbackString:
      | % fft_size = eval(get_param(gcb, 'FFTSize'));
      | % old_vec = eval(get_param(gcb, 'mult_spec'));
      | 
      | % vec = 2.*ones(1,fft_size);
      | % old_size = length(old_vec);
      | 
      | % if(old_size <= fft_size),
      | %     size = old_size;
      | % else,
      | %     size = fft_size;
      | % end
      | 
      | % for n = 1:size, vec(n) = old_vec(n); end
      | % set_param(gcb, 'mult_spec', mat2str(vec));||||||||||||specify_mult = get_param(gcb,'specify_mult');
      | visibs = get_param(gcb, 'MaskVisibilities');
      | enables = get_param(gcb, 'MaskEnables');
      | enables{14} = 'on';
      | if( strcmp(specify_mult,'off')), visibs{14} = 'off';
      | else, visibs{14} = 'on';
      | end
      | set_param(gcb, 'MaskVisibilities', visibs);
      | set_param(gcb, 'MaskEnables', enables);
      | |% vec = eval( get_param(gcb, 'mult_spec'));
      | % vec_size = length(vec);
      | % fft_size = eval( get_param(gcb, 'FFTSize'));
      | % new_vec = 2.*ones(1, fft_size);
      | 
      | % if(vec_size <= fft_size), size = vec_size;
      | % else size = fft_size;
      | % end
      | 
      | % for n=1:size
      | %    new_vec(n) = vec(n);
      | % end
      | % set_param(gcb, 'mult_spec', mat2str(new_vec));|
  fft_direct : Subsystem  AncestorBlock=casper_library/FFTs/fft_direct  mask(fft_direct){FFTSize=4, input_bit_width=18, coeff_bit_width=18, add_latency=1, mult_latency=3, bram_latency=3, quantization=Truncate, overflow=Wrap, map_tail=on, LargerFFTSize=7, StartStage=4, arch=Virtex5, opt_target=Multipliers, coeffs_bit_limit=8, specify_mult=on, mult_spec=[1 1 1 1], dsp48_adders=on}
      mask prompts: FFTSize "Size of FFT: (2^?)"; input_bit_width "Input/Output Bit Width"; coeff_bit_width "Coefficient Bit Width"; add_latency "Add Latency"; mult_latency "Mult Latency"; bram_latency "BRAM Latency"; quantization "Quantization Behavior"; overflow "Overflow Behavior"; map_tail "Map Tail of Larger FFT"; LargerFFTSize "Size of Larger FFT (2^?):"; StartStage "Start Stage of Map Tail:"; arch "Architecture"; opt_target "Optimisation Target"; coeffs_bit_limit "Number bits above which to store stage's coefficients in BRAM (2^? bits)"; specify_mult "Specify multiplier use (behavioural HDL if not specified)"; mult_spec "Multiplier specification (0=core, 1=behavioural HDL, 2=embedded) (left=1st stage)"; dsp48_adders "Use DSP48s for adders"
      mask help:
      | Implements an N-point FFT with the classic
      | butterfly structure with N inputs and N outputs
      mask init:
      | fft_direct_init(gcb,...
      |     'FFTSize', FFTSize, ...
      |     'input_bit_width', input_bit_width, ...
      |     'coeff_bit_width', coeff_bit_width, ...
      |     'add_latency', add_latency, ...
      |     'mult_latency', mult_latency, ...
      |     'bram_latency', bram_latency, ...
      |     'quantization', quantization, ...
      |     'overflow', overflow, ...
      |     'map_tail', map_tail, ...
      |     'LargerFFTSize', LargerFFTSize, ...
      |     'StartStage', StartStage, ...
      |     'arch', arch, ...
      |     'opt_target', opt_target, ...
      |     'coeffs_bit_limit', coeffs_bit_limit, ...
      |     'specify_mult', specify_mult, ...
      |     'mult_spec', mult_spec, ...
      |     'dsp48_adders', dsp48_adders);
      settings: AttributesFormatString=FFTSize=4
      mask StyleString: edit,edit,edit,edit,edit,edit,popup(Truncate|Round  (unbiased: +/- Inf)|Round  (unbiased: Even Values)),popup(Wrap|Saturate|Error),checkbox,edit,edit,popup(Virtex2Pro|Virtex5),popup(Logic|Multipliers),edit,checkbox,edit,checkbox
      mask CallbackString:
      | % fft_size = eval(get_param(gcb, 'FFTSize'));
      | % old_vec = eval(get_param(gcb, 'mult_spec'));
      | 
      | % vec = 2.*ones(1,fft_size);
      | % old_size = length(old_vec);
      | 
      | % if(old_size <= fft_size),
      | %     size = old_size;
      | % else,
      | %     size = fft_size;
      | % end
      | 
      | % for n = 1:size, vec(n) = old_vec(n); end
      | % set_param(gcb, 'mult_spec', mat2str(vec));||||||||map_tail = get_param(gcb,'map_tail');
      | visibs = get_param(gcb, 'MaskVisibilities');
      | if( strcmp(map_tail,'off')),
      |     visibs{10} = 'off';
      |     visibs{11} = 'off';
      | else,
      |     visibs{10} = 'on';
      |     visibs{11} = 'on';
      | end
      | set_param(gcb, 'MaskVisibilities', visibs);
      | ||||||specify_mult = get_param(gcb,'specify_mult');
      | visibs = get_param(gcb, 'MaskVisibilities');
      | if( strcmp(specify_mult,'off')), visibs{16} = 'off';
      | else, visibs{16} = 'on';
      | end
      | set_param(gcb, 'MaskVisibilities', visibs);
      | |% vec = eval( get_param(gcb, 'mult_spec'));
      | % vec_size = length(vec);
      | % fft_size = eval( get_param(gcb, 'FFTSize'));
      | % new_vec = 2.*ones(1, fft_size);
      | 
      | % if(vec_size <= fft_size), size = vec_size;
      | % else size = fft_size;
      | % end
      | 
      | % for n=1:size
      | %     new_vec(n) = vec(n);
      | % end
      | % set_param(gcb, 'mult_spec', mat2str(new_vec));|
  fft_unscrambler : Subsystem  AncestorBlock=casper_library/FFTs/fft_unscrambler  mask(fft_unscrambler){FFTSize=6, n_inputs=3, bram_latency=3}
      mask prompts: FFTSize "Size of FFT: (2^?)"; n_inputs "Number of Simultaneous Inputs: (2^?)"; bram_latency "BRAM Latency"
      mask init:
      | fft_unscrambler_init(gcb,...
      |     'FFTSize',FFTSize,... 
      |     'n_inputs', n_inputs,...
      |     'bram_latency', bram_latency);
      settings: AttributesFormatString=FFTSize=6, n_inputs=3
  of_or : xbsIndex_r4/Logical  logical_function=OR  inputs=5  en=off  latency=1  precision=Full  arith_type=Unsigned  n_bits=16  bin_pt=0  align_bp=on  dbl_ovrd=off  block_type=logical
  slice : xbsIndex_r4/Slice  nbits=4  boolean_output=off  mode=Lower Bit Location + Width  bit1=0  base1=MSB of Input  bit0=3  base0=LSB of Input  dbl_ovrd=off  block_type=slice
      settings: infoedit="Extracts a given range of bits from each input sample and presents it at the output.  The output type is ordinarily unsigned with binary point at zero, but can be Boolean when the slice is one bit wide.<P><P>Hardware notes: In hardware this block costs nothing."  block_version=10.1.3
  sync_out : Outport
  out0 : Outport  Port=2
  out1 : Outport  Port=3
  out2 : Outport  Port=4
  out3 : Outport  Port=5
  out4 : Outport  Port=6
  out5 : Outport  Port=7
  out6 : Outport  Port=8
  out7 : Outport  Port=9
  of : Outport  Port=10
  wires:
  in0/1 -> fft_biplex_real_4x0/1(pol1)
  in1/1 -> fft_biplex_real_4x0/2(pol2)
  in2/1 -> fft_biplex_real_4x0/3(pol3)
  in3/1 -> fft_biplex_real_4x0/4(pol4)
  shift/1 -> fft_biplex_real_4x0/6(shift), fft_biplex_real_4x1/6(shift), fft_biplex_real_4x2/6(shift), fft_biplex_real_4x3/6(shift), slice/1
  sync/1 -> fft_biplex_real_4x0/5(sync), fft_biplex_real_4x1/5(sync), fft_biplex_real_4x2/5(sync), fft_biplex_real_4x3/5(sync)
  fft_biplex_real_4x0/5(of) -> of_or/2
  in4/1 -> fft_biplex_real_4x1/1(pol1)
  in5/1 -> fft_biplex_real_4x1/2(pol2)
  in6/1 -> fft_biplex_real_4x1/3(pol3)
  in7/1 -> fft_biplex_real_4x1/4(pol4)
  fft_biplex_real_4x1/5(of) -> of_or/3
  in8/1 -> fft_biplex_real_4x2/1(pol1)
  in9/1 -> fft_biplex_real_4x2/2(pol2)
  in10/1 -> fft_biplex_real_4x2/3(pol3)
  in11/1 -> fft_biplex_real_4x2/4(pol4)
  fft_biplex_real_4x2/5(of) -> of_or/4
  in12/1 -> fft_biplex_real_4x3/1(pol1)
  in13/1 -> fft_biplex_real_4x3/2(pol2)
  in14/1 -> fft_biplex_real_4x3/3(pol3)
  in15/1 -> fft_biplex_real_4x3/4(pol4)
  fft_biplex_real_4x3/5(of) -> of_or/5
  slice/1 -> fft_direct/2(shift)
  fft_biplex_real_4x0/6(sync_out) -> fft_direct/1(sync)
  fft_biplex_real_4x0/1(pol1_out) -> fft_direct/3(in0)
  fft_biplex_real_4x0/2(pol2_out) -> fft_direct/4(in1)
  fft_biplex_real_4x0/3(pol3_out) -> fft_direct/5(in2)
  fft_biplex_real_4x0/4(pol4_out) -> fft_direct/6(in3)
  fft_biplex_real_4x1/1(pol1_out) -> fft_direct/7(in4)
  fft_biplex_real_4x1/2(pol2_out) -> fft_direct/8(in5)
  fft_biplex_real_4x1/3(pol3_out) -> fft_direct/9(in6)
  fft_biplex_real_4x1/4(pol4_out) -> fft_direct/10(in7)
  fft_biplex_real_4x2/1(pol1_out) -> fft_direct/11(in8)
  fft_biplex_real_4x2/2(pol2_out) -> fft_direct/12(in9)
  fft_biplex_real_4x2/3(pol3_out) -> fft_direct/13(in10)
  fft_biplex_real_4x2/4(pol4_out) -> fft_direct/14(in11)
  fft_biplex_real_4x3/1(pol1_out) -> fft_direct/15(in12)
  fft_biplex_real_4x3/2(pol2_out) -> fft_direct/16(in13)
  fft_biplex_real_4x3/3(pol3_out) -> fft_direct/17(in14)
  fft_biplex_real_4x3/4(pol4_out) -> fft_direct/18(in15)
  fft_direct/18(of) -> of_or/1
  of_or/1 -> of/1
  fft_direct/1(sync_out) -> fft_unscrambler/1(sync)
  fft_unscrambler/1(sync_out) -> sync_out/1
  fft_direct/2(out0) -> fft_unscrambler/2(In1)
  fft_unscrambler/2(Out1) -> out0/1
  fft_direct/3(out1) -> fft_unscrambler/3(In2)
  fft_unscrambler/3(Out2) -> out1/1
  fft_direct/4(out2) -> fft_unscrambler/4(In3)
  fft_unscrambler/4(Out3) -> out2/1
  fft_direct/5(out3) -> fft_unscrambler/5(In4)
  fft_unscrambler/5(Out4) -> out3/1
  fft_direct/6(out4) -> fft_unscrambler/6(In5)
  fft_unscrambler/6(Out5) -> out4/1
  fft_direct/7(out5) -> fft_unscrambler/7(In6)
  fft_unscrambler/7(Out6) -> out5/1
  fft_direct/8(out6) -> fft_unscrambler/8(In7)
  fft_unscrambler/8(Out7) -> out6/1
  fft_direct/9(out7) -> fft_unscrambler/9(In8)
  fft_unscrambler/9(Out8) -> out7/1
